simulink model "controller_observer_AllAgents_v5"  (Simulink 8.4)
settings: Solver=ode1  StartTime=0.0  StopTime=inf  FixedStep=60*1  RelTol=1e-3
values from neighbouring files (symbols this model uses):
  Cpw = 4180    [readepJSONout.m]
  m_max = 3    [DesignParameters.m]
  mc_MAX = 2    [DesignParameters.m]
  Tamb_additive_Sensor_fault_time = 60*60*24*(Tamb_day_ofthe_add_fault + Tamb_time_ofthe_add_fault)    (= 129600)    [faults_setting.m]
  Tamb_additive_Sensor_fault_value = 10    [faults_setting.m]
  Tamb_mult_Sensor_fault_time = 60*60*24*(Tamb_day_ofthe_mult_fault + Tamb_time_ofthe_mult_fault)    (= 129600)    [faults_setting.m]
  Tamb_mult_Sensor_fault_value = 1.1    [faults_setting.m]
  Ts = 3600/dat.Timestep.Timestep_0x20_1.number_of_timesteps_per_hour    [readepJSONout.m]
  Tst_C_additive_Sensor_fault_time = 60*60*24*(Tst_C_day_ofthe_add_fault + Tst_C_time_ofthe_add_fault)    (= 129600)    [faults_setting.m]
  Tst_C_additive_Sensor_fault_value = 10    [faults_setting.m]
  Tst_C_mult_Sensor_fault_time = 60*60*24*(Tst_C_day_ofthe_mult_fault + Tst_C_time_ofthe_mult_fault)    (= 129600)    [faults_setting.m]
  Tst_C_mult_Sensor_fault_value = 1.1    [faults_setting.m]
  Tst_H_additive_Sensor_fault_time = 60*60*24*(Tst_H_day_ofthe_add_fault + Tst_H_time_ofthe_add_fault)    (= 129600)    [faults_setting.m]
  Tst_H_additive_Sensor_fault_value = 10    [faults_setting.m]
  Tst_H_mult_Sensor_fault_time = 60*60*24*(Tst_H_day_ofthe_mult_fault + Tst_H_time_ofthe_mult_fault)    (= 129600)    [faults_setting.m]
  Tst_H_mult_Sensor_fault_value = 1.1    [faults_setting.m]
undefined symbols (not defined in the file or in its folder): building, COP, Ehp_max, fsa_E, fsa_ET, fsa_N, fsa_NT, fsa_W, fsa_WT, h_E, h_ET, h_N, h_NT, h_W, h_WT, K_NtoNT, K_NTtoN, Ke_E, Ke_ET, Ke_N, Ke_NT, Ke_WT, Km_E, Km_ET, Km_N, Km_NT, Km_W, Km_WT, Kout_N, Kout_NT, Kout_W, Kout_WT, Kst, Ksta, Kstcij, KstRef, Kw_E, Kw_ET, Kw_N, Kw_NT, Kw_W, Kw_WT
libraries: simulink x61
(part 11 of 36)

== system controller_observer_AllAgents_v5/Controller/EnableDifonifmode46 ==
  mc : Inport
  on_off : Inport  Port=2
  Constant7 : Constant  SampleTime=Ts
  Constant8 : Constant  Value=0  SampleTime=Ts
  If Action Subsystem7 : Subsystem  TreatAsAtomicUnit=on
  If Action Subsystem8 : Subsystem  TreatAsAtomicUnit=on
  If5 : If  SampleTime=Ts
  Logical Operator : Logic  AllPortsSameDT=off  OutDataTypeStr=boolean  SampleTime=Ts
  Merge3 : Merge
  enable detection : Outport
  wires:
  mc/1 -> If5/1
  If Action Subsystem8/1(Out1) -> Merge3/2
  If5/2 -> If Action Subsystem8/Action
  If5/1 -> If Action Subsystem7/Action
  Constant8/1 -> If Action Subsystem8/1(In1)
  Constant7/1 -> If Action Subsystem7/1(In1)
  If Action Subsystem7/1(Out1) -> Merge3/1
  Logical Operator/1 -> enable detection/1
  Merge3/1 -> Logical Operator/1
  on_off/1 -> Logical Operator/2

== system controller_observer_AllAgents_v5/Controller/EnableDifonifmode47 ==
  mc : Inport
  on_off : Inport  Port=2
  Constant7 : Constant  SampleTime=Ts
  Constant8 : Constant  Value=0  SampleTime=Ts
  If Action Subsystem7 : Subsystem  TreatAsAtomicUnit=on
  If Action Subsystem8 : Subsystem  TreatAsAtomicUnit=on
  If5 : If  SampleTime=Ts
  Logical Operator : Logic  AllPortsSameDT=off  OutDataTypeStr=boolean  SampleTime=Ts
  Merge3 : Merge
  enable detection : Outport
  wires:
  mc/1 -> If5/1
  If Action Subsystem8/1(Out1) -> Merge3/2
  If5/2 -> If Action Subsystem8/Action
  If5/1 -> If Action Subsystem7/Action
  Constant8/1 -> If Action Subsystem8/1(In1)
  Constant7/1 -> If Action Subsystem7/1(In1)
  If Action Subsystem7/1(Out1) -> Merge3/1
  Logical Operator/1 -> enable detection/1
  Merge3/1 -> Logical Operator/1
  on_off/1 -> Logical Operator/2

== system controller_observer_AllAgents_v5/Controller/EnableDifonifmode48 ==
  mc : Inport
  on_off : Inport  Port=2
  Constant7 : Constant  SampleTime=Ts
  Constant8 : Constant  Value=0  SampleTime=Ts
  If Action Subsystem7 : Subsystem  TreatAsAtomicUnit=on
  If Action Subsystem8 : Subsystem  TreatAsAtomicUnit=on
  If5 : If  SampleTime=Ts
  Logical Operator : Logic  AllPortsSameDT=off  OutDataTypeStr=boolean  SampleTime=Ts
  Merge3 : Merge
  enable detection : Outport
  wires:
  mc/1 -> If5/1
  If Action Subsystem8/1(Out1) -> Merge3/2
  If5/2 -> If Action Subsystem8/Action
  If5/1 -> If Action Subsystem7/Action
  Constant8/1 -> If Action Subsystem8/1(In1)
  Constant7/1 -> If Action Subsystem7/1(In1)
  If Action Subsystem7/1(Out1) -> Merge3/1
  Logical Operator/1 -> enable detection/1
  Merge3/1 -> Logical Operator/1
  on_off/1 -> Logical Operator/2

== system controller_observer_AllAgents_v5/Controller/EnableDifonifmode49 ==
  mc : Inport
  on_off : Inport  Port=2
  Constant7 : Constant  SampleTime=Ts
  Constant8 : Constant  Value=0  SampleTime=Ts
  If Action Subsystem7 : Subsystem  TreatAsAtomicUnit=on
  If Action Subsystem8 : Subsystem  TreatAsAtomicUnit=on
  If5 : If  SampleTime=Ts
  Logical Operator : Logic  AllPortsSameDT=off  OutDataTypeStr=boolean  SampleTime=Ts
  Merge3 : Merge
  enable detection : Outport
  wires:
  mc/1 -> If5/1
  If Action Subsystem8/1(Out1) -> Merge3/2
  If5/2 -> If Action Subsystem8/Action
  If5/1 -> If Action Subsystem7/Action
  Constant8/1 -> If Action Subsystem8/1(In1)
  Constant7/1 -> If Action Subsystem7/1(In1)
  If Action Subsystem7/1(Out1) -> Merge3/1
  Logical Operator/1 -> enable detection/1
  Merge3/1 -> Logical Operator/1
  on_off/1 -> Logical Operator/2

== system controller_observer_AllAgents_v5/Controller/EnableDifonifmode5 ==
  mc : Inport
  on_off : Inport  Port=2
  Constant7 : Constant  SampleTime=Ts
  Constant8 : Constant  Value=0  SampleTime=Ts
  If Action Subsystem7 : Subsystem  TreatAsAtomicUnit=on
  If Action Subsystem8 : Subsystem  TreatAsAtomicUnit=on
  If5 : If  SampleTime=Ts
  Logical Operator : Logic  AllPortsSameDT=off  OutDataTypeStr=boolean  SampleTime=Ts
  Merge3 : Merge
  enable detection : Outport
  wires:
  mc/1 -> If5/1
  If Action Subsystem8/1(Out1) -> Merge3/2
  If5/2 -> If Action Subsystem8/Action
  If5/1 -> If Action Subsystem7/Action
  Constant8/1 -> If Action Subsystem8/1(In1)
  Constant7/1 -> If Action Subsystem7/1(In1)
  If Action Subsystem7/1(Out1) -> Merge3/1
  Logical Operator/1 -> enable detection/1
  Merge3/1 -> Logical Operator/1
  on_off/1 -> Logical Operator/2

== system controller_observer_AllAgents_v5/Controller/EnableDifonifmode50 ==
  mc : Inport
  on_off : Inport  Port=2
  Constant7 : Constant  SampleTime=Ts
  Constant8 : Constant  Value=0  SampleTime=Ts
  If Action Subsystem7 : Subsystem  TreatAsAtomicUnit=on
  If Action Subsystem8 : Subsystem  TreatAsAtomicUnit=on
  If5 : If  SampleTime=Ts
  Logical Operator : Logic  AllPortsSameDT=off  OutDataTypeStr=boolean  SampleTime=Ts
  Merge3 : Merge
  enable detection : Outport
  wires:
  mc/1 -> If5/1
  If Action Subsystem8/1(Out1) -> Merge3/2
  If5/2 -> If Action Subsystem8/Action
  If5/1 -> If Action Subsystem7/Action
  Constant8/1 -> If Action Subsystem8/1(In1)
  Constant7/1 -> If Action Subsystem7/1(In1)
  If Action Subsystem7/1(Out1) -> Merge3/1
  Logical Operator/1 -> enable detection/1
  Merge3/1 -> Logical Operator/1
  on_off/1 -> Logical Operator/2

== system controller_observer_AllAgents_v5/Controller/EnableDifonifmode6 ==
  mc : Inport
  on_off : Inport  Port=2
  Constant7 : Constant  SampleTime=Ts
  Constant8 : Constant  Value=0  SampleTime=Ts
  If Action Subsystem7 : Subsystem  TreatAsAtomicUnit=on
  If Action Subsystem8 : Subsystem  TreatAsAtomicUnit=on
  If5 : If  SampleTime=Ts
  Logical Operator : Logic  AllPortsSameDT=off  OutDataTypeStr=boolean  SampleTime=Ts
  Merge3 : Merge
  enable detection : Outport
  wires:
  mc/1 -> If5/1
  If Action Subsystem8/1(Out1) -> Merge3/2
  If5/2 -> If Action Subsystem8/Action
  If5/1 -> If Action Subsystem7/Action
  Constant8/1 -> If Action Subsystem8/1(In1)
  Constant7/1 -> If Action Subsystem7/1(In1)
  If Action Subsystem7/1(Out1) -> Merge3/1
  Logical Operator/1 -> enable detection/1
  Merge3/1 -> Logical Operator/1
  on_off/1 -> Logical Operator/2

== system controller_observer_AllAgents_v5/Controller/EnableDifonifmode7 ==
  mc : Inport
  on_off : Inport  Port=2
  Constant7 : Constant  SampleTime=Ts
  Constant8 : Constant  Value=0  SampleTime=Ts
  If Action Subsystem7 : Subsystem  TreatAsAtomicUnit=on
  If Action Subsystem8 : Subsystem  TreatAsAtomicUnit=on
  If5 : If  SampleTime=Ts
  Logical Operator : Logic  AllPortsSameDT=off  OutDataTypeStr=boolean  SampleTime=Ts
  Merge3 : Merge
  enable detection : Outport
  wires:
  mc/1 -> If5/1
  If Action Subsystem8/1(Out1) -> Merge3/2
  If5/2 -> If Action Subsystem8/Action
  If5/1 -> If Action Subsystem7/Action
  Constant8/1 -> If Action Subsystem8/1(In1)
  Constant7/1 -> If Action Subsystem7/1(In1)
  If Action Subsystem7/1(Out1) -> Merge3/1
  Logical Operator/1 -> enable detection/1
  Merge3/1 -> Logical Operator/1
  on_off/1 -> Logical Operator/2

== system controller_observer_AllAgents_v5/Controller/EnableDifonifmode8 ==
  mc : Inport
  on_off : Inport  Port=2
  Constant7 : Constant  SampleTime=Ts
  Constant8 : Constant  Value=0  SampleTime=Ts
  If Action Subsystem7 : Subsystem  TreatAsAtomicUnit=on
  If Action Subsystem8 : Subsystem  TreatAsAtomicUnit=on
  If5 : If  SampleTime=Ts
  Logical Operator : Logic  AllPortsSameDT=off  OutDataTypeStr=boolean  SampleTime=Ts
  Merge3 : Merge
  enable detection : Outport
  wires:
  mc/1 -> If5/1
  If Action Subsystem8/1(Out1) -> Merge3/2
  If5/2 -> If Action Subsystem8/Action
  If5/1 -> If Action Subsystem7/Action
  Constant8/1 -> If Action Subsystem8/1(In1)
  Constant7/1 -> If Action Subsystem7/1(In1)
  If Action Subsystem7/1(Out1) -> Merge3/1
  Logical Operator/1 -> enable detection/1
  Merge3/1 -> Logical Operator/1
  on_off/1 -> Logical Operator/2

== system controller_observer_AllAgents_v5/Controller/EnableDifonifmode9 ==
  mc : Inport
  on_off : Inport  Port=2
  Constant7 : Constant  SampleTime=Ts
  Constant8 : Constant  Value=0  SampleTime=Ts
  If Action Subsystem7 : Subsystem  TreatAsAtomicUnit=on
  If Action Subsystem8 : Subsystem  TreatAsAtomicUnit=on
  If5 : If  SampleTime=Ts
  Logical Operator : Logic  AllPortsSameDT=off  OutDataTypeStr=boolean  SampleTime=Ts
  Merge3 : Merge
  enable detection : Outport
  wires:
  mc/1 -> If5/1
  If Action Subsystem8/1(Out1) -> Merge3/2
  If5/2 -> If Action Subsystem8/Action
  If5/1 -> If Action Subsystem7/Action
  Constant8/1 -> If Action Subsystem8/1(In1)
  Constant7/1 -> If Action Subsystem7/1(In1)
  If Action Subsystem7/1(Out1) -> Merge3/1
  Logical Operator/1 -> enable detection/1
  Merge3/1 -> Logical Operator/1
  on_off/1 -> Logical Operator/2

== system controller_observer_AllAgents_v5/Controller/Fault_Tamb ==
  In : Inport
  Add : Sum  Inputs=+++  SampleTime=Ts
  Add2 : Sum  Inputs=+-  SampleTime=Ts
  Clock : Clock
  Constant : Constant  Value=Tamb_mult_Sensor_fault_time  SampleTime=Ts
  Constant2 : Constant  Value=building.zone(9).z_incr_Sensor_fault_time  SampleTime=Ts
  Dot Product : DotProduct  SampleTime=Ts  InputSameDT=on  OutDataTypeStr=Inherit: Inherit via internal rule
  Fcn : Fcn  Expr=1-0.99^(u)  SampleTime=Ts
  Gain : Gain  Gain=Tamb_mult_Sensor_fault_value  SampleTime=Ts
  Gain1 : Gain  Gain=building.zone(9).z_incr_Sensor_fault_value  SampleTime=Ts
  Gain3 : Gain  Gain=1/Ts  SampleTime=Ts
  If : If  NumInputs=2  IfExpression=u1 >= u2  SampleTime=Ts
  If Action Subsystem : Subsystem  TreatAsAtomicUnit=on
  If Action Subsystem1 : Subsystem  TreatAsAtomicUnit=on
  Merge : Merge
  Step : Step  Time=Tamb_additive_Sensor_fault_time  After=Tamb_additive_Sensor_fault_value  SampleTime=Ts
  Step1 : Step  Time=building.zone(9).z_incr_Sensor_fault_time  SampleTime=Ts
  Out : Outport
  wires:
  If/2 -> If Action Subsystem1/Action
  Add/1 -> Out/1
  Step/1 -> Add/1
  Merge/1 -> Add/2
  If/1 -> If Action Subsystem/Action
  If Action Subsystem/1(Out1) -> Merge/1
  In/1 -> If Action Subsystem1/1(In1), Gain/1
  If Action Subsystem1/1(Out1) -> Merge/2
  Gain/1 -> If Action Subsystem/1(In1)
  Constant/1 -> If/2
  Clock/1 -> Add2/1, If/1  "k"
  Dot Product/1 -> Fcn/1
  Step1/1 -> Dot Product/2
  Constant2/1 -> Add2/2
  Fcn/1 -> Gain1/1
  Add2/1 -> Gain3/1
  Gain3/1 -> Dot Product/1
  Gain1/1 -> Add/3

== system controller_observer_AllAgents_v5/Controller/Fault_Tc_Bath_Cooling ==
  In : Inport
  Add : Sum  Inputs=+++  SampleTime=Ts
  Add2 : Sum  Inputs=+-  SampleTime=Ts
  Clock : Clock
  Constant : Constant  Value=building.zone(1).c_C_mult_Sensor_fault_time  SampleTime=Ts
  Constant2 : Constant  Value=building.zone(1).c_C_incr_Sensor_fault_time  SampleTime=Ts
  Dot Product : DotProduct  SampleTime=Ts  InputSameDT=on  OutDataTypeStr=Inherit: Inherit via internal rule
  Fcn : Fcn  Expr=1-0.99^(u)  SampleTime=Ts
  Gain : Gain  Gain=building.zone(1).c_C_mult_Sensor_fault_value  SampleTime=Ts
  Gain1 : Gain  Gain=building.zone(1).c_C_incr_Sensor_fault_value  SampleTime=Ts
  Gain3 : Gain  Gain=1/Ts  SampleTime=Ts
  If : If  NumInputs=2  IfExpression=u1 >= u2  SampleTime=Ts
  If Action Subsystem : Subsystem  TreatAsAtomicUnit=on
  If Action Subsystem1 : Subsystem  TreatAsAtomicUnit=on
  Merge : Merge
  Step : Step  Time=building.zone(1).c_C_additive_Sensor_fault_time  After=building.zone(1).c_C_additive_Sensor_fault_value  SampleTime=Ts
  Step1 : Step  Time=building.zone(1).c_C_incr_Sensor_fault_time  SampleTime=Ts
  Out : Outport
  wires:
  If/2 -> If Action Subsystem1/Action
  Add/1 -> Out/1
  Step/1 -> Add/1
  Merge/1 -> Add/2
  If/1 -> If Action Subsystem/Action
  If Action Subsystem/1(Out1) -> Merge/1
  In/1 -> If Action Subsystem1/1(In1), Gain/1
  If Action Subsystem1/1(Out1) -> Merge/2
  Gain/1 -> If Action Subsystem/1(In1)
  Constant/1 -> If/2
  Clock/1 -> Add2/1, If/1  "k"
  Dot Product/1 -> Fcn/1
  Step1/1 -> Dot Product/2
  Constant2/1 -> Add2/2
  Fcn/1 -> Gain1/1
  Add2/1 -> Gain3/1
  Gain3/1 -> Dot Product/1
  Gain1/1 -> Add/3

== system controller_observer_AllAgents_v5/Controller/Fault_Tc_Bath_Heating ==
  In : Inport
  Add : Sum  Inputs=+++  SampleTime=Ts
  Add2 : Sum  Inputs=+-  SampleTime=Ts
  Clock : Clock
  Constant : Constant  Value=building.zone(1).c_H_mult_Sensor_fault_time  SampleTime=Ts
  Constant2 : Constant  Value=building.zone(1).c_H_incr_Sensor_fault_time  SampleTime=Ts
  Dot Product : DotProduct  SampleTime=Ts  InputSameDT=on  OutDataTypeStr=Inherit: Inherit via internal rule
  Fcn : Fcn  Expr=1-0.99^(u)  SampleTime=Ts
  Gain : Gain  Gain=building.zone(1).c_H_mult_Sensor_fault_value  SampleTime=Ts
  Gain1 : Gain  Gain=building.zone(1).c_H_incr_Sensor_fault_value  SampleTime=Ts
  Gain3 : Gain  Gain=1/Ts  SampleTime=Ts
  If : If  NumInputs=2  IfExpression=u1 >= u2  SampleTime=Ts
  If Action Subsystem : Subsystem  TreatAsAtomicUnit=on
  If Action Subsystem1 : Subsystem  TreatAsAtomicUnit=on
  Merge : Merge
  Step : Step  Time=building.zone(1).c_H_additive_Sensor_fault_time  After=building.zone(1).c_H_additive_Sensor_fault_value  SampleTime=Ts
  Step1 : Step  Time=building.zone(1).c_H_incr_Sensor_fault_time  SampleTime=Ts
  Out : Outport
  wires:
  If/2 -> If Action Subsystem1/Action
  Add/1 -> Out/1
  Step/1 -> Add/1
  Merge/1 -> Add/2
  If/1 -> If Action Subsystem/Action
  If Action Subsystem/1(Out1) -> Merge/1
  In/1 -> If Action Subsystem1/1(In1), Gain/1
  If Action Subsystem1/1(Out1) -> Merge/2
  Gain/1 -> If Action Subsystem/1(In1)
  Constant/1 -> If/2
  Clock/1 -> Add2/1, If/1  "k"
  Dot Product/1 -> Fcn/1
  Step1/1 -> Dot Product/2
  Constant2/1 -> Add2/2
  Fcn/1 -> Gain1/1
  Add2/1 -> Gain3/1
  Gain3/1 -> Dot Product/1
  Gain1/1 -> Add/3

== system controller_observer_AllAgents_v5/Controller/Fault_Tc_Cafeteria_Cooling ==
  In : Inport
  Add : Sum  Inputs=+++  SampleTime=Ts
  Add2 : Sum  Inputs=+-  SampleTime=Ts
  Clock : Clock
  Constant : Constant  Value=building.zone(2).c_C_mult_Sensor_fault_time  SampleTime=Ts
  Constant2 : Constant  Value=building.zone(2).c_C_incr_Sensor_fault_time  SampleTime=Ts
  Dot Product : DotProduct  SampleTime=Ts  InputSameDT=on  OutDataTypeStr=Inherit: Inherit via internal rule
  Fcn : Fcn  Expr=1-0.99^(u)  SampleTime=Ts
  Gain : Gain  Gain=building.zone(2).c_C_mult_Sensor_fault_value  SampleTime=Ts
  Gain1 : Gain  Gain=building.zone(2).c_C_incr_Sensor_fault_value  SampleTime=Ts
  Gain3 : Gain  Gain=1/Ts  SampleTime=Ts
  If : If  NumInputs=2  IfExpression=u1 >= u2  SampleTime=Ts
  If Action Subsystem : Subsystem  TreatAsAtomicUnit=on
  If Action Subsystem1 : Subsystem  TreatAsAtomicUnit=on
  Merge : Merge
  Step : Step  Time=building.zone(2).c_C_additive_Sensor_fault_time  After=building.zone(2).c_C_additive_Sensor_fault_value  SampleTime=Ts
  Step1 : Step  Time=building.zone(2).c_C_incr_Sensor_fault_time  SampleTime=Ts
  Out : Outport
  wires:
  If/2 -> If Action Subsystem1/Action
  Add/1 -> Out/1
  Step/1 -> Add/1
  Merge/1 -> Add/2
  If/1 -> If Action Subsystem/Action
  If Action Subsystem/1(Out1) -> Merge/1
  In/1 -> If Action Subsystem1/1(In1), Gain/1
  If Action Subsystem1/1(Out1) -> Merge/2
  Gain/1 -> If Action Subsystem/1(In1)
  Constant/1 -> If/2
  Clock/1 -> Add2/1, If/1  "k"
  Dot Product/1 -> Fcn/1
  Step1/1 -> Dot Product/2
  Constant2/1 -> Add2/2
  Fcn/1 -> Gain1/1
  Add2/1 -> Gain3/1
  Gain3/1 -> Dot Product/1
  Gain1/1 -> Add/3

== system controller_observer_AllAgents_v5/Controller/Fault_Tc_Cafeteria_Heating ==
  In : Inport
  Add : Sum  Inputs=+++  SampleTime=Ts
  Add2 : Sum  Inputs=+-  SampleTime=Ts
  Clock : Clock
  Constant : Constant  Value=building.zone(2).c_H_mult_Sensor_fault_time  SampleTime=Ts
  Constant2 : Constant  Value=building.zone(2).c_H_incr_Sensor_fault_time  SampleTime=Ts
  Dot Product : DotProduct  SampleTime=Ts  InputSameDT=on  OutDataTypeStr=Inherit: Inherit via internal rule
  Fcn : Fcn  Expr=1-0.99^(u)  SampleTime=Ts
  Gain : Gain  Gain=building.zone(2).c_H_mult_Sensor_fault_value  SampleTime=Ts
  Gain1 : Gain  Gain=building.zone(2).c_H_incr_Sensor_fault_value  SampleTime=Ts
  Gain3 : Gain  Gain=1/Ts  SampleTime=Ts
  If : If  NumInputs=2  IfExpression=u1 >= u2  SampleTime=Ts
  If Action Subsystem : Subsystem  TreatAsAtomicUnit=on
  If Action Subsystem1 : Subsystem  TreatAsAtomicUnit=on
  Merge : Merge
  Step : Step  Time=building.zone(2).c_H_additive_Sensor_fault_time  After=building.zone(2).c_H_additive_Sensor_fault_value  SampleTime=Ts
  Step1 : Step  Time=building.zone(2).c_H_incr_Sensor_fault_time  SampleTime=Ts
  Out : Outport
  wires:
  If/2 -> If Action Subsystem1/Action
  Add/1 -> Out/1
  Step/1 -> Add/1
  Merge/1 -> Add/2
  If/1 -> If Action Subsystem/Action
  If Action Subsystem/1(Out1) -> Merge/1
  In/1 -> If Action Subsystem1/1(In1), Gain/1
  If Action Subsystem1/1(Out1) -> Merge/2
  Gain/1 -> If Action Subsystem/1(In1)
  Constant/1 -> If/2
  Clock/1 -> Add2/1, If/1  "k"
  Dot Product/1 -> Fcn/1
  Step1/1 -> Dot Product/2
  Constant2/1 -> Add2/2
  Fcn/1 -> Gain1/1
  Add2/1 -> Gain3/1
  Gain3/1 -> Dot Product/1
  Gain1/1 -> Add/3

== system controller_observer_AllAgents_v5/Controller/Fault_Tc_ComputerClass_Cooling ==
  In : Inport
  Add : Sum  Inputs=+++  SampleTime=Ts
  Add2 : Sum  Inputs=+-  SampleTime=Ts
  Clock : Clock
  Constant : Constant  Value=building.zone(3).c_C_mult_Sensor_fault_time  SampleTime=Ts
  Constant2 : Constant  Value=building.zone(3).c_C_incr_Sensor_fault_time  SampleTime=Ts
  Dot Product : DotProduct  SampleTime=Ts  InputSameDT=on  OutDataTypeStr=Inherit: Inherit via internal rule
  Fcn : Fcn  Expr=1-0.99^(u)  SampleTime=Ts
  Gain : Gain  Gain=building.zone(3).c_C_mult_Sensor_fault_value  SampleTime=Ts
  Gain1 : Gain  Gain=building.zone(3).c_C_incr_Sensor_fault_value  SampleTime=Ts
  Gain3 : Gain  Gain=1/Ts  SampleTime=Ts
  If : If  NumInputs=2  IfExpression=u1 >= u2  SampleTime=Ts
  If Action Subsystem : Subsystem  TreatAsAtomicUnit=on
  If Action Subsystem1 : Subsystem  TreatAsAtomicUnit=on
  Merge : Merge
  Step : Step  Time=building.zone(3).c_C_additive_Sensor_fault_time  After=building.zone(3).c_C_additive_Sensor_fault_value  SampleTime=Ts
  Step1 : Step  Time=building.zone(3).c_C_incr_Sensor_fault_time  SampleTime=Ts
  Out : Outport
  wires:
  If/2 -> If Action Subsystem1/Action
  Add/1 -> Out/1
  Step/1 -> Add/1
  Merge/1 -> Add/2
  If/1 -> If Action Subsystem/Action
  If Action Subsystem/1(Out1) -> Merge/1
  In/1 -> If Action Subsystem1/1(In1), Gain/1
  If Action Subsystem1/1(Out1) -> Merge/2
  Gain/1 -> If Action Subsystem/1(In1)
  Constant/1 -> If/2
  Clock/1 -> Add2/1, If/1  "k"
  Dot Product/1 -> Fcn/1
  Step1/1 -> Dot Product/2
  Constant2/1 -> Add2/2
  Fcn/1 -> Gain1/1
  Add2/1 -> Gain3/1
  Gain3/1 -> Dot Product/1
  Gain1/1 -> Add/3

== system controller_observer_AllAgents_v5/Controller/Fault_Tc_ComputerClass_Heating ==
  In : Inport
  Add : Sum  Inputs=+++  SampleTime=Ts
  Add2 : Sum  Inputs=+-  SampleTime=Ts
  Clock : Clock
  Constant : Constant  Value=building.zone(3).c_H_mult_Sensor_fault_time  SampleTime=Ts
  Constant2 : Constant  Value=building.zone(3).c_H_incr_Sensor_fault_time  SampleTime=Ts
  Dot Product : DotProduct  SampleTime=Ts  InputSameDT=on  OutDataTypeStr=Inherit: Inherit via internal rule
  Fcn : Fcn  Expr=1-0.99^(u)  SampleTime=Ts
  Gain : Gain  Gain=building.zone(3).c_H_mult_Sensor_fault_value  SampleTime=Ts
  Gain1 : Gain  Gain=building.zone(3).c_H_incr_Sensor_fault_value  SampleTime=Ts
  Gain3 : Gain  Gain=1/Ts  SampleTime=Ts
  If : If  NumInputs=2  IfExpression=u1 >= u2  SampleTime=Ts
  If Action Subsystem : Subsystem  TreatAsAtomicUnit=on
  If Action Subsystem1 : Subsystem  TreatAsAtomicUnit=on
  Merge : Merge
  Step : Step  Time=building.zone(3).c_H_additive_Sensor_fault_time  After=building.zone(3).c_H_additive_Sensor_fault_value  SampleTime=Ts
  Step1 : Step  Time=building.zone(3).c_H_incr_Sensor_fault_time  SampleTime=Ts
  Out : Outport
  wires:
  If/2 -> If Action Subsystem1/Action
  Add/1 -> Out/1
  Step/1 -> Add/1
  Merge/1 -> Add/2
  If/1 -> If Action Subsystem/Action
  If Action Subsystem/1(Out1) -> Merge/1
  In/1 -> If Action Subsystem1/1(In1), Gain/1
  If Action Subsystem1/1(Out1) -> Merge/2
  Gain/1 -> If Action Subsystem/1(In1)
  Constant/1 -> If/2
  Clock/1 -> Add2/1, If/1  "k"
  Dot Product/1 -> Fcn/1
  Step1/1 -> Dot Product/2
  Constant2/1 -> Add2/2
  Fcn/1 -> Gain1/1
  Add2/1 -> Gain3/1
  Gain3/1 -> Dot Product/1
  Gain1/1 -> Add/3

== system controller_observer_AllAgents_v5/Controller/Fault_Tc_CornerClass1Pod1_Cooling ==
  In : Inport
  Add : Sum  Inputs=+++  SampleTime=Ts
  Add2 : Sum  Inputs=+-  SampleTime=Ts
  Clock : Clock
  Constant : Constant  Value=building.zone(4).c_C_mult_Sensor_fault_time  SampleTime=Ts
  Constant2 : Constant  Value=building.zone(4).c_C_incr_Sensor_fault_time  SampleTime=Ts
  Dot Product : DotProduct  SampleTime=Ts  InputSameDT=on  OutDataTypeStr=Inherit: Inherit via internal rule
  Fcn : Fcn  Expr=1-0.99^(u)  SampleTime=Ts
  Gain : Gain  Gain=building.zone(4).c_C_mult_Sensor_fault_value  SampleTime=Ts
  Gain1 : Gain  Gain=building.zone(4).c_C_incr_Sensor_fault_value  SampleTime=Ts
  Gain3 : Gain  Gain=1/Ts  SampleTime=Ts
  If : If  NumInputs=2  IfExpression=u1 >= u2  SampleTime=Ts
  If Action Subsystem : Subsystem  TreatAsAtomicUnit=on
  If Action Subsystem1 : Subsystem  TreatAsAtomicUnit=on
  Merge : Merge
  Step : Step  Time=building.zone(4).c_C_additive_Sensor_fault_time  After=building.zone(4).c_C_additive_Sensor_fault_value  SampleTime=Ts
  Step1 : Step  Time=building.zone(4).c_C_incr_Sensor_fault_time  SampleTime=Ts
  Out : Outport
  wires:
  If/2 -> If Action Subsystem1/Action
  Add/1 -> Out/1
  Step/1 -> Add/1
  Merge/1 -> Add/2
  If/1 -> If Action Subsystem/Action
  If Action Subsystem/1(Out1) -> Merge/1
  In/1 -> If Action Subsystem1/1(In1), Gain/1
  If Action Subsystem1/1(Out1) -> Merge/2
  Gain/1 -> If Action Subsystem/1(In1)
  Constant/1 -> If/2
  Clock/1 -> Add2/1, If/1  "k"
  Dot Product/1 -> Fcn/1
  Step1/1 -> Dot Product/2
  Constant2/1 -> Add2/2
  Fcn/1 -> Gain1/1
  Add2/1 -> Gain3/1
  Gain3/1 -> Dot Product/1
  Gain1/1 -> Add/3

== system controller_observer_AllAgents_v5/Controller/Fault_Tc_CornerClass1Pod1_Heating ==
  In : Inport
  Add : Sum  Inputs=+++  SampleTime=Ts
  Add2 : Sum  Inputs=+-  SampleTime=Ts
  Clock : Clock
  Constant : Constant  Value=building.zone(4).c_H_mult_Sensor_fault_time  SampleTime=Ts
  Constant2 : Constant  Value=building.zone(4).c_H_incr_Sensor_fault_time  SampleTime=Ts
  Dot Product : DotProduct  SampleTime=Ts  InputSameDT=on  OutDataTypeStr=Inherit: Inherit via internal rule
  Fcn : Fcn  Expr=1-0.99^(u)  SampleTime=Ts
  Gain : Gain  Gain=building.zone(4).c_H_mult_Sensor_fault_value  SampleTime=Ts
  Gain1 : Gain  Gain=building.zone(4).c_H_incr_Sensor_fault_value  SampleTime=Ts
  Gain3 : Gain  Gain=1/Ts  SampleTime=Ts
  If : If  NumInputs=2  IfExpression=u1 >= u2  SampleTime=Ts
  If Action Subsystem : Subsystem  TreatAsAtomicUnit=on
  If Action Subsystem1 : Subsystem  TreatAsAtomicUnit=on
  Merge : Merge
  Step : Step  Time=building.zone(4).c_H_additive_Sensor_fault_time  After=building.zone(4).c_H_additive_Sensor_fault_value  SampleTime=Ts
  Step1 : Step  Time=building.zone(4).c_H_incr_Sensor_fault_time  SampleTime=Ts
  Out : Outport
  wires:
  If/2 -> If Action Subsystem1/Action
  Add/1 -> Out/1
  Step/1 -> Add/1
  Merge/1 -> Add/2
  If/1 -> If Action Subsystem/Action
  If Action Subsystem/1(Out1) -> Merge/1
  In/1 -> If Action Subsystem1/1(In1), Gain/1
  If Action Subsystem1/1(Out1) -> Merge/2
  Gain/1 -> If Action Subsystem/1(In1)
  Constant/1 -> If/2
  Clock/1 -> Add2/1, If/1  "k"
  Dot Product/1 -> Fcn/1
  Step1/1 -> Dot Product/2
  Constant2/1 -> Add2/2
  Fcn/1 -> Gain1/1
  Add2/1 -> Gain3/1
  Gain3/1 -> Dot Product/1
  Gain1/1 -> Add/3

== system controller_observer_AllAgents_v5/Controller/Fault_Tc_CornerClass1Pod2_Cooling ==
  In : Inport
  Add : Sum  Inputs=+++  SampleTime=Ts
  Add2 : Sum  Inputs=+-  SampleTime=Ts
  Clock : Clock
  Constant : Constant  Value=building.zone(5).c_C_mult_Sensor_fault_time  SampleTime=Ts
  Constant2 : Constant  Value=building.zone(5).c_C_incr_Sensor_fault_time  SampleTime=Ts
  Dot Product : DotProduct  SampleTime=Ts  InputSameDT=on  OutDataTypeStr=Inherit: Inherit via internal rule
  Fcn : Fcn  Expr=1-0.99^(u)  SampleTime=Ts
  Gain : Gain  Gain=building.zone(5).c_C_mult_Sensor_fault_value  SampleTime=Ts
  Gain1 : Gain  Gain=building.zone(5).c_C_incr_Sensor_fault_value  SampleTime=Ts
  Gain3 : Gain  Gain=1/Ts  SampleTime=Ts
  If : If  NumInputs=2  IfExpression=u1 >= u2  SampleTime=Ts
  If Action Subsystem : Subsystem  TreatAsAtomicUnit=on
  If Action Subsystem1 : Subsystem  TreatAsAtomicUnit=on
  Merge : Merge
  Step : Step  Time=building.zone(5).c_C_additive_Sensor_fault_time  After=building.zone(5).c_C_additive_Sensor_fault_value  SampleTime=Ts
  Step1 : Step  Time=building.zone(5).c_C_incr_Sensor_fault_time  SampleTime=Ts
  Out : Outport
  wires:
  If/2 -> If Action Subsystem1/Action
  Add/1 -> Out/1
  Step/1 -> Add/1
  Merge/1 -> Add/2
  If/1 -> If Action Subsystem/Action
  If Action Subsystem/1(Out1) -> Merge/1
  In/1 -> If Action Subsystem1/1(In1), Gain/1
  If Action Subsystem1/1(Out1) -> Merge/2
  Gain/1 -> If Action Subsystem/1(In1)
  Constant/1 -> If/2
  Clock/1 -> Add2/1, If/1  "k"
  Dot Product/1 -> Fcn/1
  Step1/1 -> Dot Product/2
  Constant2/1 -> Add2/2
  Fcn/1 -> Gain1/1
  Add2/1 -> Gain3/1
  Gain3/1 -> Dot Product/1
  Gain1/1 -> Add/3

== system controller_observer_AllAgents_v5/Controller/Fault_Tc_CornerClass1Pod2_Heating ==
  In : Inport
  Add : Sum  Inputs=+++  SampleTime=Ts
  Add2 : Sum  Inputs=+-  SampleTime=Ts
  Clock : Clock
  Constant : Constant  Value=building.zone(5).c_H_mult_Sensor_fault_time  SampleTime=Ts
  Constant2 : Constant  Value=building.zone(5).c_H_incr_Sensor_fault_time  SampleTime=Ts
  Dot Product : DotProduct  SampleTime=Ts  InputSameDT=on  OutDataTypeStr=Inherit: Inherit via internal rule
  Fcn : Fcn  Expr=1-0.99^(u)  SampleTime=Ts
  Gain : Gain  Gain=building.zone(5).c_H_mult_Sensor_fault_value  SampleTime=Ts
  Gain1 : Gain  Gain=building.zone(5).c_H_incr_Sensor_fault_value  SampleTime=Ts
  Gain3 : Gain  Gain=1/Ts  SampleTime=Ts
  If : If  NumInputs=2  IfExpression=u1 >= u2  SampleTime=Ts
  If Action Subsystem : Subsystem  TreatAsAtomicUnit=on
  If Action Subsystem1 : Subsystem  TreatAsAtomicUnit=on
  Merge : Merge
  Step : Step  Time=building.zone(5).c_H_additive_Sensor_fault_time  After=building.zone(5).c_H_additive_Sensor_fault_value  SampleTime=Ts
  Step1 : Step  Time=building.zone(5).c_H_incr_Sensor_fault_time  SampleTime=Ts
  Out : Outport
  wires:
  If/2 -> If Action Subsystem1/Action
  Add/1 -> Out/1
  Step/1 -> Add/1
  Merge/1 -> Add/2
  If/1 -> If Action Subsystem/Action
  If Action Subsystem/1(Out1) -> Merge/1
  In/1 -> If Action Subsystem1/1(In1), Gain/1
  If Action Subsystem1/1(Out1) -> Merge/2
  Gain/1 -> If Action Subsystem/1(In1)
  Constant/1 -> If/2
  Clock/1 -> Add2/1, If/1  "k"
  Dot Product/1 -> Fcn/1
  Step1/1 -> Dot Product/2
  Constant2/1 -> Add2/2
  Fcn/1 -> Gain1/1
  Add2/1 -> Gain3/1
  Gain3/1 -> Dot Product/1
  Gain1/1 -> Add/3

== system controller_observer_AllAgents_v5/Controller/Fault_Tc_CornerClass1Pod3_Cooling ==
  In : Inport
  Add : Sum  Inputs=+++  SampleTime=Ts
  Add2 : Sum  Inputs=+-  SampleTime=Ts
  Clock : Clock
  Constant : Constant  Value=building.zone(6).c_C_mult_Sensor_fault_time  SampleTime=Ts
  Constant2 : Constant  Value=building.zone(6).c_C_incr_Sensor_fault_time  SampleTime=Ts
  Dot Product : DotProduct  SampleTime=Ts  InputSameDT=on  OutDataTypeStr=Inherit: Inherit via internal rule
  Fcn : Fcn  Expr=1-0.99^(u)  SampleTime=Ts
  Gain : Gain  Gain=building.zone(6).c_C_mult_Sensor_fault_value  SampleTime=Ts
  Gain1 : Gain  Gain=building.zone(6).c_C_incr_Sensor_fault_value  SampleTime=Ts
  Gain3 : Gain  Gain=1/Ts  SampleTime=Ts
  If : If  NumInputs=2  IfExpression=u1 >= u2  SampleTime=Ts
  If Action Subsystem : Subsystem  TreatAsAtomicUnit=on
  If Action Subsystem1 : Subsystem  TreatAsAtomicUnit=on
  Merge : Merge
  Step : Step  Time=building.zone(6).c_C_additive_Sensor_fault_time  After=building.zone(6).c_C_additive_Sensor_fault_value  SampleTime=Ts
  Step1 : Step  Time=building.zone(6).c_C_incr_Sensor_fault_time  SampleTime=Ts
  Out : Outport
  wires:
  If/2 -> If Action Subsystem1/Action
  Add/1 -> Out/1
  Step/1 -> Add/1
  Merge/1 -> Add/2
  If/1 -> If Action Subsystem/Action
  If Action Subsystem/1(Out1) -> Merge/1
  In/1 -> If Action Subsystem1/1(In1), Gain/1
  If Action Subsystem1/1(Out1) -> Merge/2
  Gain/1 -> If Action Subsystem/1(In1)
  Constant/1 -> If/2
  Clock/1 -> Add2/1, If/1  "k"
  Dot Product/1 -> Fcn/1
  Step1/1 -> Dot Product/2
  Constant2/1 -> Add2/2
  Fcn/1 -> Gain1/1
  Add2/1 -> Gain3/1
  Gain3/1 -> Dot Product/1
  Gain1/1 -> Add/3

== system controller_observer_AllAgents_v5/Controller/Fault_Tc_CornerClass1Pod3_Heating ==
  In : Inport
  Add : Sum  Inputs=+++  SampleTime=Ts
  Add2 : Sum  Inputs=+-  SampleTime=Ts
  Clock : Clock
  Constant : Constant  Value=building.zone(6).c_H_mult_Sensor_fault_time  SampleTime=Ts
  Constant2 : Constant  Value=building.zone(6).c_H_incr_Sensor_fault_time  SampleTime=Ts
  Dot Product : DotProduct  SampleTime=Ts  InputSameDT=on  OutDataTypeStr=Inherit: Inherit via internal rule
  Fcn : Fcn  Expr=1-0.99^(u)  SampleTime=Ts
  Gain : Gain  Gain=building.zone(6).c_H_mult_Sensor_fault_value  SampleTime=Ts
  Gain1 : Gain  Gain=building.zone(6).c_H_incr_Sensor_fault_value  SampleTime=Ts
  Gain3 : Gain  Gain=1/Ts  SampleTime=Ts
  If : If  NumInputs=2  IfExpression=u1 >= u2  SampleTime=Ts
  If Action Subsystem : Subsystem  TreatAsAtomicUnit=on
  If Action Subsystem1 : Subsystem  TreatAsAtomicUnit=on
  Merge : Merge
  Step : Step  Time=building.zone(6).c_H_additive_Sensor_fault_time  After=building.zone(6).c_H_additive_Sensor_fault_value  SampleTime=Ts
  Step1 : Step  Time=building.zone(6).c_H_incr_Sensor_fault_time  SampleTime=Ts
  Out : Outport
  wires:
  If/2 -> If Action Subsystem1/Action
  Add/1 -> Out/1
  Step/1 -> Add/1
  Merge/1 -> Add/2
  If/1 -> If Action Subsystem/Action
  If Action Subsystem/1(Out1) -> Merge/1
  In/1 -> If Action Subsystem1/1(In1), Gain/1
  If Action Subsystem1/1(Out1) -> Merge/2
  Gain/1 -> If Action Subsystem/1(In1)
  Constant/1 -> If/2
  Clock/1 -> Add2/1, If/1  "k"
  Dot Product/1 -> Fcn/1
  Step1/1 -> Dot Product/2
  Constant2/1 -> Add2/2
  Fcn/1 -> Gain1/1
  Add2/1 -> Gain3/1
  Gain3/1 -> Dot Product/1
  Gain1/1 -> Add/3

== system controller_observer_AllAgents_v5/Controller/Fault_Tc_CornerClass2Pod1_Cooling ==
  In : Inport
  Add : Sum  Inputs=+++  SampleTime=Ts
  Add2 : Sum  Inputs=+-  SampleTime=Ts
  Clock : Clock
  Constant : Constant  Value=building.zone(7).c_C_mult_Sensor_fault_time  SampleTime=Ts
  Constant2 : Constant  Value=building.zone(7).c_C_incr_Sensor_fault_time  SampleTime=Ts
  Dot Product : DotProduct  SampleTime=Ts  InputSameDT=on  OutDataTypeStr=Inherit: Inherit via internal rule
  Fcn : Fcn  Expr=1-0.99^(u)  SampleTime=Ts
  Gain : Gain  Gain=building.zone(7).c_C_mult_Sensor_fault_value  SampleTime=Ts
  Gain1 : Gain  Gain=building.zone(7).c_C_incr_Sensor_fault_value  SampleTime=Ts
  Gain3 : Gain  Gain=1/Ts  SampleTime=Ts
  If : If  NumInputs=2  IfExpression=u1 >= u2  SampleTime=Ts
  If Action Subsystem : Subsystem  TreatAsAtomicUnit=on
  If Action Subsystem1 : Subsystem  TreatAsAtomicUnit=on
  Merge : Merge
  Step : Step  Time=building.zone(7).c_C_additive_Sensor_fault_time  After=building.zone(7).c_C_additive_Sensor_fault_value  SampleTime=Ts
  Step1 : Step  Time=building.zone(7).c_C_incr_Sensor_fault_time  SampleTime=Ts
  Out : Outport
  wires:
  If/2 -> If Action Subsystem1/Action
  Add/1 -> Out/1
  Step/1 -> Add/1
  Merge/1 -> Add/2
  If/1 -> If Action Subsystem/Action
  If Action Subsystem/1(Out1) -> Merge/1
  In/1 -> If Action Subsystem1/1(In1), Gain/1
  If Action Subsystem1/1(Out1) -> Merge/2
  Gain/1 -> If Action Subsystem/1(In1)
  Constant/1 -> If/2
  Clock/1 -> Add2/1, If/1  "k"
  Dot Product/1 -> Fcn/1
  Step1/1 -> Dot Product/2
  Constant2/1 -> Add2/2
  Fcn/1 -> Gain1/1
  Add2/1 -> Gain3/1
  Gain3/1 -> Dot Product/1
  Gain1/1 -> Add/3

== system controller_observer_AllAgents_v5/Controller/Fault_Tc_CornerClass2Pod1_Heating ==
  In : Inport
  Add : Sum  Inputs=+++  SampleTime=Ts
  Add2 : Sum  Inputs=+-  SampleTime=Ts
  Clock : Clock
  Constant : Constant  Value=building.zone(7).c_H_mult_Sensor_fault_time  SampleTime=Ts
  Constant2 : Constant  Value=building.zone(7).c_H_incr_Sensor_fault_time  SampleTime=Ts
  Dot Product : DotProduct  SampleTime=Ts  InputSameDT=on  OutDataTypeStr=Inherit: Inherit via internal rule
  Fcn : Fcn  Expr=1-0.99^(u)  SampleTime=Ts
  Gain : Gain  Gain=building.zone(7).c_H_mult_Sensor_fault_value  SampleTime=Ts
  Gain1 : Gain  Gain=building.zone(7).c_H_incr_Sensor_fault_value  SampleTime=Ts
  Gain3 : Gain  Gain=1/Ts  SampleTime=Ts
  If : If  NumInputs=2  IfExpression=u1 >= u2  SampleTime=Ts
  If Action Subsystem : Subsystem  TreatAsAtomicUnit=on
  If Action Subsystem1 : Subsystem  TreatAsAtomicUnit=on
  Merge : Merge
  Step : Step  Time=building.zone(7).c_H_additive_Sensor_fault_time  After=building.zone(7).c_H_additive_Sensor_fault_value  SampleTime=Ts
  Step1 : Step  Time=building.zone(7).c_H_incr_Sensor_fault_time  SampleTime=Ts
  Out : Outport
  wires:
  If/2 -> If Action Subsystem1/Action
  Add/1 -> Out/1
  Step/1 -> Add/1
  Merge/1 -> Add/2
  If/1 -> If Action Subsystem/Action
  If Action Subsystem/1(Out1) -> Merge/1
  In/1 -> If Action Subsystem1/1(In1), Gain/1
  If Action Subsystem1/1(Out1) -> Merge/2
  Gain/1 -> If Action Subsystem/1(In1)
  Constant/1 -> If/2
  Clock/1 -> Add2/1, If/1  "k"
  Dot Product/1 -> Fcn/1
  Step1/1 -> Dot Product/2
  Constant2/1 -> Add2/2
  Fcn/1 -> Gain1/1
  Add2/1 -> Gain3/1
  Gain3/1 -> Dot Product/1
  Gain1/1 -> Add/3

== system controller_observer_AllAgents_v5/Controller/Fault_Tc_CornerClass2Pod2_Cooling ==
  In : Inport
  Add : Sum  Inputs=+++  SampleTime=Ts
  Add2 : Sum  Inputs=+-  SampleTime=Ts
  Clock : Clock
  Constant : Constant  Value=building.zone(8).c_C_mult_Sensor_fault_time  SampleTime=Ts
  Constant2 : Constant  Value=building.zone(8).c_C_incr_Sensor_fault_time  SampleTime=Ts
  Dot Product : DotProduct  SampleTime=Ts  InputSameDT=on  OutDataTypeStr=Inherit: Inherit via internal rule
  Fcn : Fcn  Expr=1-0.99^(u)  SampleTime=Ts
  Gain : Gain  Gain=building.zone(8).c_C_mult_Sensor_fault_value  SampleTime=Ts
  Gain1 : Gain  Gain=building.zone(8).c_C_incr_Sensor_fault_value  SampleTime=Ts
  Gain3 : Gain  Gain=1/Ts  SampleTime=Ts
  If : If  NumInputs=2  IfExpression=u1 >= u2  SampleTime=Ts
  If Action Subsystem : Subsystem  TreatAsAtomicUnit=on
  If Action Subsystem1 : Subsystem  TreatAsAtomicUnit=on
  Merge : Merge
  Step : Step  Time=building.zone(8).c_C_additive_Sensor_fault_time  After=building.zone(8).c_C_additive_Sensor_fault_value  SampleTime=Ts
  Step1 : Step  Time=building.zone(8).c_C_incr_Sensor_fault_time  SampleTime=Ts
  Out : Outport
  wires:
  If/2 -> If Action Subsystem1/Action
  Add/1 -> Out/1
  Step/1 -> Add/1
  Merge/1 -> Add/2
  If/1 -> If Action Subsystem/Action
  If Action Subsystem/1(Out1) -> Merge/1
  In/1 -> If Action Subsystem1/1(In1), Gain/1
  If Action Subsystem1/1(Out1) -> Merge/2
  Gain/1 -> If Action Subsystem/1(In1)
  Constant/1 -> If/2
  Clock/1 -> Add2/1, If/1  "k"
  Dot Product/1 -> Fcn/1
  Step1/1 -> Dot Product/2
  Constant2/1 -> Add2/2
  Fcn/1 -> Gain1/1
  Add2/1 -> Gain3/1
  Gain3/1 -> Dot Product/1
  Gain1/1 -> Add/3

== system controller_observer_AllAgents_v5/Controller/Fault_Tc_CornerClass2Pod2_Heating ==
  In : Inport
  Add : Sum  Inputs=+++  SampleTime=Ts
  Add2 : Sum  Inputs=+-  SampleTime=Ts
  Clock : Clock
  Constant : Constant  Value=building.zone(8).c_H_mult_Sensor_fault_time  SampleTime=Ts
  Constant2 : Constant  Value=building.zone(8).c_H_incr_Sensor_fault_time  SampleTime=Ts
  Dot Product : DotProduct  SampleTime=Ts  InputSameDT=on  OutDataTypeStr=Inherit: Inherit via internal rule
  Fcn : Fcn  Expr=1-0.99^(u)  SampleTime=Ts
  Gain : Gain  Gain=building.zone(8).c_H_mult_Sensor_fault_value  SampleTime=Ts
  Gain1 : Gain  Gain=building.zone(8).c_H_incr_Sensor_fault_value  SampleTime=Ts
  Gain3 : Gain  Gain=1/Ts  SampleTime=Ts
  If : If  NumInputs=2  IfExpression=u1 >= u2  SampleTime=Ts
  If Action Subsystem : Subsystem  TreatAsAtomicUnit=on
  If Action Subsystem1 : Subsystem  TreatAsAtomicUnit=on
  Merge : Merge
  Step : Step  Time=building.zone(8).c_H_additive_Sensor_fault_time  After=building.zone(8).c_H_additive_Sensor_fault_value  SampleTime=Ts
  Step1 : Step  Time=building.zone(8).c_H_incr_Sensor_fault_time  SampleTime=Ts
  Out : Outport
  wires:
  If/2 -> If Action Subsystem1/Action
  Add/1 -> Out/1
  Step/1 -> Add/1
  Merge/1 -> Add/2
  If/1 -> If Action Subsystem/Action
  If Action Subsystem/1(Out1) -> Merge/1
  In/1 -> If Action Subsystem1/1(In1), Gain/1
  If Action Subsystem1/1(Out1) -> Merge/2
  Gain/1 -> If Action Subsystem/1(In1)
  Constant/1 -> If/2
  Clock/1 -> Add2/1, If/1  "k"
  Dot Product/1 -> Fcn/1
  Step1/1 -> Dot Product/2
  Constant2/1 -> Add2/2
  Fcn/1 -> Gain1/1
  Add2/1 -> Gain3/1
  Gain3/1 -> Dot Product/1
  Gain1/1 -> Add/3

== system controller_observer_AllAgents_v5/Controller/Fault_Tc_CornerClass2Pod3_Cooling ==
  In : Inport
  Add : Sum  Inputs=+++  SampleTime=Ts
  Add2 : Sum  Inputs=+-  SampleTime=Ts
  Clock : Clock
  Constant : Constant  Value=building.zone(9).c_C_mult_Sensor_fault_time  SampleTime=Ts
  Constant2 : Constant  Value=building.zone(9).c_C_incr_Sensor_fault_time  SampleTime=Ts
  Dot Product : DotProduct  SampleTime=Ts  InputSameDT=on  OutDataTypeStr=Inherit: Inherit via internal rule
  Fcn : Fcn  Expr=1-0.99^(u)  SampleTime=Ts
  Gain : Gain  Gain=building.zone(9).c_C_mult_Sensor_fault_value  SampleTime=Ts
  Gain1 : Gain  Gain=building.zone(9).c_C_incr_Sensor_fault_value  SampleTime=Ts
  Gain3 : Gain  Gain=1/Ts  SampleTime=Ts
  If : If  NumInputs=2  IfExpression=u1 >= u2  SampleTime=Ts
  If Action Subsystem : Subsystem  TreatAsAtomicUnit=on
  If Action Subsystem1 : Subsystem  TreatAsAtomicUnit=on
  Merge : Merge
  Step : Step  Time=building.zone(9).c_C_additive_Sensor_fault_time  After=building.zone(9).c_C_additive_Sensor_fault_value  SampleTime=Ts
  Step1 : Step  Time=building.zone(9).c_C_incr_Sensor_fault_time  SampleTime=Ts
  Out : Outport
  wires:
  If/2 -> If Action Subsystem1/Action
  Add/1 -> Out/1
  Step/1 -> Add/1
  Merge/1 -> Add/2
  If/1 -> If Action Subsystem/Action
  If Action Subsystem/1(Out1) -> Merge/1
  In/1 -> If Action Subsystem1/1(In1), Gain/1
  If Action Subsystem1/1(Out1) -> Merge/2
  Gain/1 -> If Action Subsystem/1(In1)
  Constant/1 -> If/2
  Clock/1 -> Add2/1, If/1  "k"
  Dot Product/1 -> Fcn/1
  Step1/1 -> Dot Product/2
  Constant2/1 -> Add2/2
  Fcn/1 -> Gain1/1
  Add2/1 -> Gain3/1
  Gain3/1 -> Dot Product/1
  Gain1/1 -> Add/3

== system controller_observer_AllAgents_v5/Controller/Fault_Tc_CornerClass2Pod3_Heating ==
  In : Inport
  Add : Sum  Inputs=+++  SampleTime=Ts
  Add2 : Sum  Inputs=+-  SampleTime=Ts
  Clock : Clock
  Constant : Constant  Value=building.zone(9).c_H_mult_Sensor_fault_time  SampleTime=Ts
  Constant2 : Constant  Value=building.zone(9).c_H_incr_Sensor_fault_time  SampleTime=Ts
  Dot Product : DotProduct  SampleTime=Ts  InputSameDT=on  OutDataTypeStr=Inherit: Inherit via internal rule
  Fcn : Fcn  Expr=1-0.99^(u)  SampleTime=Ts
  Gain : Gain  Gain=building.zone(9).c_H_mult_Sensor_fault_value  SampleTime=Ts
  Gain1 : Gain  Gain=building.zone(9).c_H_incr_Sensor_fault_value  SampleTime=Ts
  Gain3 : Gain  Gain=1/Ts  SampleTime=Ts
  If : If  NumInputs=2  IfExpression=u1 >= u2  SampleTime=Ts
  If Action Subsystem : Subsystem  TreatAsAtomicUnit=on
  If Action Subsystem1 : Subsystem  TreatAsAtomicUnit=on
  Merge : Merge
  Step : Step  Time=building.zone(9).c_H_additive_Sensor_fault_time  After=building.zone(9).c_H_additive_Sensor_fault_value  SampleTime=Ts
  Step1 : Step  Time=building.zone(9).c_H_incr_Sensor_fault_time  SampleTime=Ts
  Out : Outport
  wires:
  If/2 -> If Action Subsystem1/Action
  Add/1 -> Out/1
  Step/1 -> Add/1
  Merge/1 -> Add/2
  If/1 -> If Action Subsystem/Action
  If Action Subsystem/1(Out1) -> Merge/1
  In/1 -> If Action Subsystem1/1(In1), Gain/1
  If Action Subsystem1/1(Out1) -> Merge/2
  Gain/1 -> If Action Subsystem/1(In1)
  Constant/1 -> If/2
  Clock/1 -> Add2/1, If/1  "k"
  Dot Product/1 -> Fcn/1
  Step1/1 -> Dot Product/2
  Constant2/1 -> Add2/2
  Fcn/1 -> Gain1/1
  Add2/1 -> Gain3/1
  Gain3/1 -> Dot Product/1
  Gain1/1 -> Add/3

== system controller_observer_AllAgents_v5/Controller/Fault_Tc_CorridorPod1_Cooling ==
  In : Inport
  Add : Sum  Inputs=+++  SampleTime=Ts
  Add2 : Sum  Inputs=+-  SampleTime=Ts
  Clock : Clock
  Constant : Constant  Value=building.zone(10).c_C_mult_Sensor_fault_time  SampleTime=Ts
  Constant2 : Constant  Value=building.zone(10).c_C_incr_Sensor_fault_time  SampleTime=Ts
  Dot Product : DotProduct  SampleTime=Ts  InputSameDT=on  OutDataTypeStr=Inherit: Inherit via internal rule
  Fcn : Fcn  Expr=1-0.99^(u)  SampleTime=Ts
  Gain : Gain  Gain=building.zone(10).c_C_mult_Sensor_fault_value  SampleTime=Ts
  Gain1 : Gain  Gain=building.zone(10).c_C_incr_Sensor_fault_value  SampleTime=Ts
  Gain3 : Gain  Gain=1/Ts  SampleTime=Ts
  If : If  NumInputs=2  IfExpression=u1 >= u2  SampleTime=Ts
  If Action Subsystem : Subsystem  TreatAsAtomicUnit=on
  If Action Subsystem1 : Subsystem  TreatAsAtomicUnit=on
  Merge : Merge
  Step : Step  Time=building.zone(10).c_C_additive_Sensor_fault_time  After=building.zone(10).c_C_additive_Sensor_fault_value  SampleTime=Ts
  Step1 : Step  Time=building.zone(10).c_C_incr_Sensor_fault_time  SampleTime=Ts
  Out : Outport
  wires:
  If/2 -> If Action Subsystem1/Action
  Add/1 -> Out/1
  Step/1 -> Add/1
  Merge/1 -> Add/2
  If/1 -> If Action Subsystem/Action
  If Action Subsystem/1(Out1) -> Merge/1
  In/1 -> If Action Subsystem1/1(In1), Gain/1
  If Action Subsystem1/1(Out1) -> Merge/2
  Gain/1 -> If Action Subsystem/1(In1)
  Constant/1 -> If/2
  Clock/1 -> Add2/1, If/1  "k"
  Dot Product/1 -> Fcn/1
  Step1/1 -> Dot Product/2
  Constant2/1 -> Add2/2
  Fcn/1 -> Gain1/1
  Add2/1 -> Gain3/1
  Gain3/1 -> Dot Product/1
  Gain1/1 -> Add/3

== system controller_observer_AllAgents_v5/Controller/Fault_Tc_CorridorPod1_Heating ==
  In : Inport
  Add : Sum  Inputs=+++  SampleTime=Ts
  Add2 : Sum  Inputs=+-  SampleTime=Ts
  Clock : Clock
  Constant : Constant  Value=building.zone(10).c_H_mult_Sensor_fault_time  SampleTime=Ts
  Constant2 : Constant  Value=building.zone(10).c_H_incr_Sensor_fault_time  SampleTime=Ts
  Dot Product : DotProduct  SampleTime=Ts  InputSameDT=on  OutDataTypeStr=Inherit: Inherit via internal rule
  Fcn : Fcn  Expr=1-0.99^(u)  SampleTime=Ts
  Gain : Gain  Gain=building.zone(10).c_H_mult_Sensor_fault_value  SampleTime=Ts
  Gain1 : Gain  Gain=building.zone(10).c_H_incr_Sensor_fault_value  SampleTime=Ts
  Gain3 : Gain  Gain=1/Ts  SampleTime=Ts
  If : If  NumInputs=2  IfExpression=u1 >= u2  SampleTime=Ts
  If Action Subsystem : Subsystem  TreatAsAtomicUnit=on
  If Action Subsystem1 : Subsystem  TreatAsAtomicUnit=on
  Merge : Merge
  Step : Step  Time=building.zone(10).c_H_additive_Sensor_fault_time  After=building.zone(10).c_H_additive_Sensor_fault_value  SampleTime=Ts
  Step1 : Step  Time=building.zone(10).c_H_incr_Sensor_fault_time  SampleTime=Ts
  Out : Outport
  wires:
  If/2 -> If Action Subsystem1/Action
  Add/1 -> Out/1
  Step/1 -> Add/1
  Merge/1 -> Add/2
  If/1 -> If Action Subsystem/Action
  If Action Subsystem/1(Out1) -> Merge/1
  In/1 -> If Action Subsystem1/1(In1), Gain/1
  If Action Subsystem1/1(Out1) -> Merge/2
  Gain/1 -> If Action Subsystem/1(In1)
  Constant/1 -> If/2
  Clock/1 -> Add2/1, If/1  "k"
  Dot Product/1 -> Fcn/1
  Step1/1 -> Dot Product/2
  Constant2/1 -> Add2/2
  Fcn/1 -> Gain1/1
  Add2/1 -> Gain3/1
  Gain3/1 -> Dot Product/1
  Gain1/1 -> Add/3

== system controller_observer_AllAgents_v5/Controller/Fault_Tc_CorridorPod2_Cooling ==
  In : Inport
  Add : Sum  Inputs=+++  SampleTime=Ts
  Add2 : Sum  Inputs=+-  SampleTime=Ts
  Clock : Clock
  Constant : Constant  Value=building.zone(11).c_C_mult_Sensor_fault_time  SampleTime=Ts
  Constant2 : Constant  Value=building.zone(11).c_C_incr_Sensor_fault_time  SampleTime=Ts
  Dot Product : DotProduct  SampleTime=Ts  InputSameDT=on  OutDataTypeStr=Inherit: Inherit via internal rule
  Fcn : Fcn  Expr=1-0.99^(u)  SampleTime=Ts
  Gain : Gain  Gain=building.zone(11).c_C_mult_Sensor_fault_value  SampleTime=Ts
  Gain1 : Gain  Gain=building.zone(11).c_C_incr_Sensor_fault_value  SampleTime=Ts
  Gain3 : Gain  Gain=1/Ts  SampleTime=Ts
  If : If  NumInputs=2  IfExpression=u1 >= u2  SampleTime=Ts
  If Action Subsystem : Subsystem  TreatAsAtomicUnit=on
  If Action Subsystem1 : Subsystem  TreatAsAtomicUnit=on
  Merge : Merge
  Step : Step  Time=building.zone(11).c_C_additive_Sensor_fault_time  After=building.zone(11).c_C_additive_Sensor_fault_value  SampleTime=Ts
  Step1 : Step  Time=building.zone(11).c_C_incr_Sensor_fault_time  SampleTime=Ts
  Out : Outport
  wires:
  If/2 -> If Action Subsystem1/Action
  Add/1 -> Out/1
  Step/1 -> Add/1
  Merge/1 -> Add/2
  If/1 -> If Action Subsystem/Action
  If Action Subsystem/1(Out1) -> Merge/1
  In/1 -> If Action Subsystem1/1(In1), Gain/1
  If Action Subsystem1/1(Out1) -> Merge/2
  Gain/1 -> If Action Subsystem/1(In1)
  Constant/1 -> If/2
  Clock/1 -> Add2/1, If/1  "k"
  Dot Product/1 -> Fcn/1
  Step1/1 -> Dot Product/2
  Constant2/1 -> Add2/2
  Fcn/1 -> Gain1/1
  Add2/1 -> Gain3/1
  Gain3/1 -> Dot Product/1
  Gain1/1 -> Add/3

== system controller_observer_AllAgents_v5/Controller/Fault_Tc_CorridorPod2_Heating ==
  In : Inport
  Add : Sum  Inputs=+++  SampleTime=Ts
  Add2 : Sum  Inputs=+-  SampleTime=Ts
  Clock : Clock
  Constant : Constant  Value=building.zone(11).c_H_mult_Sensor_fault_time  SampleTime=Ts
  Constant2 : Constant  Value=building.zone(11).c_H_incr_Sensor_fault_time  SampleTime=Ts
  Dot Product : DotProduct  SampleTime=Ts  InputSameDT=on  OutDataTypeStr=Inherit: Inherit via internal rule
  Fcn : Fcn  Expr=1-0.99^(u)  SampleTime=Ts
  Gain : Gain  Gain=building.zone(11).c_H_mult_Sensor_fault_value  SampleTime=Ts
  Gain1 : Gain  Gain=building.zone(11).c_H_incr_Sensor_fault_value  SampleTime=Ts
  Gain3 : Gain  Gain=1/Ts  SampleTime=Ts
  If : If  NumInputs=2  IfExpression=u1 >= u2  SampleTime=Ts
  If Action Subsystem : Subsystem  TreatAsAtomicUnit=on
  If Action Subsystem1 : Subsystem  TreatAsAtomicUnit=on
  Merge : Merge
  Step : Step  Time=building.zone(11).c_H_additive_Sensor_fault_time  After=building.zone(11).c_H_additive_Sensor_fault_value  SampleTime=Ts
  Step1 : Step  Time=building.zone(11).c_H_incr_Sensor_fault_time  SampleTime=Ts
  Out : Outport
  wires:
  If/2 -> If Action Subsystem1/Action
  Add/1 -> Out/1
  Step/1 -> Add/1
  Merge/1 -> Add/2
  If/1 -> If Action Subsystem/Action
  If Action Subsystem/1(Out1) -> Merge/1
  In/1 -> If Action Subsystem1/1(In1), Gain/1
  If Action Subsystem1/1(Out1) -> Merge/2
  Gain/1 -> If Action Subsystem/1(In1)
  Constant/1 -> If/2
  Clock/1 -> Add2/1, If/1  "k"
  Dot Product/1 -> Fcn/1
  Step1/1 -> Dot Product/2
  Constant2/1 -> Add2/2
  Fcn/1 -> Gain1/1
  Add2/1 -> Gain3/1
  Gain3/1 -> Dot Product/1
  Gain1/1 -> Add/3
